simulink model "testDirect"  (Simulink 7.6)
settings: Solver=ode45  StartTime=0.0  StopTime=1400.0  RelTol=1e-3
libraries: xbsIndex_r4 x2187, casper_library x117, monroe_library x15, xps_library x1
(part 3 of 20)

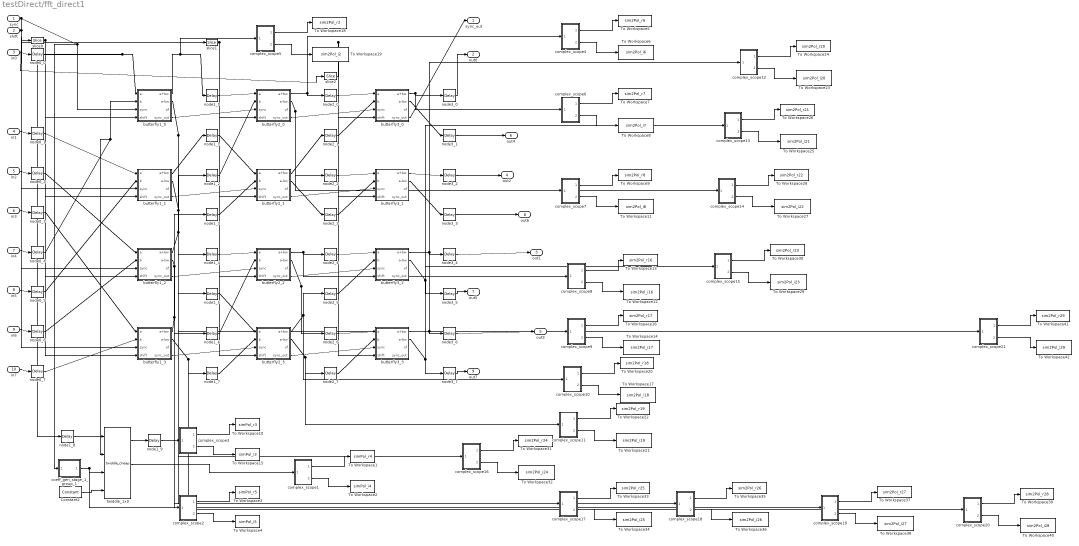
== system testDirect/fft_direct1 ==
  sync : Inport
  shift : Inport  Port=2
  in0 : Inport  Port=3
  in1 : Inport  Port=4
  in2 : Inport  Port=5
  in3 : Inport  Port=6
  in4 : Inport  Port=7
  in5 : Inport  Port=8
  in6 : Inport  Port=9
  in7 : Inport  Port=10
  Constant2 : xbsIndex_r4/Constant  arith_type=Signed (2's comp)  const=0.2  n_bits=18  bin_pt=17  explicit_period=on  period=1  equ=P=C  opselect=C  inp2=PCIN>>17  opr=+  inp1=P  carry=CIN  dbl_ovrd=off  block_type=constant
  To Workspace1 : ToWorkspace  VariableName=simPol_r4  SampleTime=-1  SaveFormat=Structure
  To Workspace10 : ToWorkspace  VariableName=simPol_r3  SampleTime=-1  SaveFormat=Structure
  To Workspace11 : ToWorkspace  VariableName=sim2Pol_i8  SampleTime=-1  SaveFormat=Structure
  To Workspace12 : ToWorkspace  VariableName=sim2Pol_i16  SampleTime=-1  SaveFormat=Structure
  To Workspace13 : ToWorkspace  VariableName=sim2Pol_r16  SampleTime=-1  SaveFormat=Structure
  To Workspace14 : ToWorkspace  VariableName=sim2Pol_i17  SampleTime=-1  SaveFormat=Structure
  To Workspace15 : ToWorkspace  VariableName=simPol_i3  SampleTime=-1  SaveFormat=Structure
  To Workspace16 : ToWorkspace  VariableName=sim2Pol_r17  SampleTime=-1  SaveFormat=Structure
  To Workspace17 : ToWorkspace  VariableName=sim2Pol_i18  SampleTime=-1  SaveFormat=Structure
  To Workspace18 : ToWorkspace  VariableName=sim2Pol_r2  SampleTime=-1  SaveFormat=Structure
  To Workspace19 : ToWorkspace  VariableName=sim2Pol_i2  SampleTime=-1  SaveFormat=Structure
  To Workspace2 : ToWorkspace  VariableName=simPol_i4  SampleTime=-1  SaveFormat=Structure
  To Workspace20 : ToWorkspace  VariableName=sim2Pol_r18  SampleTime=-1  SaveFormat=Structure
  To Workspace21 : ToWorkspace  VariableName=sim2Pol_i19  SampleTime=-1  SaveFormat=Structure
  To Workspace22 : ToWorkspace  VariableName=sim2Pol_r19  SampleTime=-1  SaveFormat=Structure
  To Workspace23 : ToWorkspace  VariableName=sim2Pol_i20  SampleTime=-1  SaveFormat=Structure
  To Workspace24 : ToWorkspace  VariableName=sim2Pol_r20  SampleTime=-1  SaveFormat=Structure
  To Workspace25 : ToWorkspace  VariableName=sim2Pol_i21  SampleTime=-1  SaveFormat=Structure
  To Workspace26 : ToWorkspace  VariableName=sim2Pol_r21  SampleTime=-1  SaveFormat=Structure
  To Workspace27 : ToWorkspace  VariableName=sim2Pol_i22  SampleTime=-1  SaveFormat=Structure
  To Workspace28 : ToWorkspace  VariableName=sim2Pol_r22  SampleTime=-1  SaveFormat=Structure
  To Workspace29 : ToWorkspace  VariableName=sim2Pol_i23  SampleTime=-1  SaveFormat=Structure
  To Workspace3 : ToWorkspace  VariableName=simPol_r5  SampleTime=-1  SaveFormat=Structure
  To Workspace30 : ToWorkspace  VariableName=sim2Pol_r23  SampleTime=-1  SaveFormat=Structure
  To Workspace31 : ToWorkspace  VariableName=sim2Pol_r24  SampleTime=-1  SaveFormat=Structure
  To Workspace32 : ToWorkspace  VariableName=sim2Pol_i24  SampleTime=-1  SaveFormat=Structure
  To Workspace33 : ToWorkspace  VariableName=sim2Pol_r25  SampleTime=-1  SaveFormat=Structure
  To Workspace34 : ToWorkspace  VariableName=sim2Pol_i25  SampleTime=-1  SaveFormat=Structure
  To Workspace35 : ToWorkspace  VariableName=sim2Pol_r26  SampleTime=-1  SaveFormat=Structure
  To Workspace36 : ToWorkspace  VariableName=sim2Pol_i26  SampleTime=-1  SaveFormat=Structure
  To Workspace37 : ToWorkspace  VariableName=sim2Pol_r27  SampleTime=-1  SaveFormat=Structure
  To Workspace38 : ToWorkspace  VariableName=sim2Pol_i27  SampleTime=-1  SaveFormat=Structure
  To Workspace39 : ToWorkspace  VariableName=sim2Pol_r28  SampleTime=-1  SaveFormat=Structure
  To Workspace4 : ToWorkspace  VariableName=simPol_i5  SampleTime=-1  SaveFormat=Structure
  To Workspace40 : ToWorkspace  VariableName=sim2Pol_i28  SampleTime=-1  SaveFormat=Structure
  To Workspace41 : ToWorkspace  VariableName=sim2Pol_r29  SampleTime=-1  SaveFormat=Structure
  To Workspace42 : ToWorkspace  VariableName=sim2Pol_i29  SampleTime=-1  SaveFormat=Structure
  To Workspace5 : ToWorkspace  VariableName=sim2Pol_r6  SampleTime=-1  SaveFormat=Structure
  To Workspace6 : ToWorkspace  VariableName=sim2Pol_i6  SampleTime=-1  SaveFormat=Structure
  To Workspace7 : ToWorkspace  VariableName=sim2Pol_r7  SampleTime=-1  SaveFormat=Structure
  To Workspace8 : ToWorkspace  VariableName=sim2Pol_i7  SampleTime=-1  SaveFormat=Structure
  To Workspace9 : ToWorkspace  VariableName=sim2Pol_r8  SampleTime=-1  SaveFormat=Structure
  butterfly1_0 : Subsystem  mask(butterfly_direct){biplex=off, FFTSize=8, Coeffs=getfield( getfield( get_param( gcb,'UserData' ), 'parameters'),'Coeffs'), coeff_bit_width=18, StepPeriod=0, bram_latency=2, coeffs_bram=on, input_bit_width=18, add_latency=2, mult_latency=3, quantization=Truncate, overflow=Wrap, arch=Virtex5, opt_target=Logic, use_hdl=on, use_embedded=off, dsp48_adders=on, shift=1, output_bit_width=18}
      mask prompts: biplex "Used in biplex FFT"; FFTSize "Size of FFT: (2^?)"; Coeffs "Coefficients: (0 to 2^(FFTSize-1)-1)"; coeff_bit_width "Coefficient Bit Width"; StepPeriod "Coefficient Step Period: (2^?)"; bram_latency "BRAM Latency"; coeffs_bram "Implement coefficients in BRAM"; input_bit_width "Input/Output Bit Width:"; add_latency "Add Latency"; mult_latency "Mult Latency"; quantization "Quantization Behavior"; overflow "Overflow Behavior"; arch "Architecture"; opt_target "Optimisation target"; use_hdl "Use behavioural HDL for multipliers"; use_embedded "Use embedded multipliers"; dsp48_adders "Use DSP48s for adders"; shift "Downshift binary point? (1 or 0)"; output_bit_width "Output Bit Width"
      mask help:
      | implements adders efficiently using 2 dsp48e slices, manually instatiated
      |
      | Update Log:
      | 08/05, 5:45 PM PST: inputs and outputs are registered
      | 08/24, 23:22 PST: 'shift' hard coded
      mask init:
      | butterfly_direct_init(gcb,...
      |               'biplex', biplex,...
      |               'FFTSize', FFTSize,...
      |               'Coeffs', Coeffs,...
      |               'coeff_bit_width', coeff_bit_width,...
      |               'StepPeriod', StepPeriod,...
      |               'bram_latency', bram_latency,...
      |               'coeffs_bram', coeffs_bram,...
      |               'input_bit_width', input_bit_width,...
      |               'output_bit_width', output_bit_width,...
      |               'add_latency', add_latency,...
      |               'mult_latency', mult_latency,...
      |               'quantization', quantization,...
      |               'overflow', overflow,...
      |               'arch', arch,...
      |               'opt_target', opt_target,...
      |               'use_hdl', use_hdl,...
      |               'use_embedded', use_embedded,...
      |               'dsp48_adders', dsp48_adders);
      settings: AttributesFormatString=FFTSize=8, coeff_bit_width=18
      mask StyleString: checkbox,edit,edit,edit,edit,edit,checkbox,edit,edit,edit,popup(Truncate|Round  (unbiased: +/- Inf)|Round  (unbiased: Even Values)),popup(Wrap|Saturate|Error),popup(Virtex2Pro|Virtex5),popup(Logic|Multipliers),checkbox,checkbox,checkbox,edit,edit
      mask CallbackString:
      | ||coeffs = eval(get_param(gcb,'Coeffs'));
      | visibs = get_param(gcb,'MaskVisibilities');
      | if length(coeffs) == 1,
      |     visibs{5} = 'off'; visibs{6} = 'off';
      |     visibs{7} = 'off';
      | else
      |     visibs{5} = 'on';
      |     visibs{6} = 'on'; visibs{7} = 'on';
      | end
      | set_param(gcb, 'MaskVisibilities', visibs);||||||||||||use_hdl = get_param(gcb,'use_hdl');
      | visibs = get_param(gcb, 'MaskVisibilities');
      | if( strcmp(use_hdl,'on')),
      |     set_param(gcb, 'use_embedded','off');
      |     visibs{16} = 'off';
      | else,
      |     visibs{16} = 'on';
      | end
      |     set_param(gcb, 'MaskVisibilities',visibs);||||
  butterfly1_1 : Subsystem  mask(butterfly_direct){biplex=off, FFTSize=8, Coeffs=getfield( getfield( get_param( gcb,'UserData' ), 'parameters'),'Coeffs'), coeff_bit_width=18, StepPeriod=0, bram_latency=2, coeffs_bram=on, input_bit_width=18, add_latency=2, mult_latency=3, quantization=Truncate, overflow=Wrap, arch=Virtex5, opt_target=Logic, use_hdl=on, use_embedded=off, dsp48_adders=on, shift=1, output_bit_width=18}
      mask prompts: biplex "Used in biplex FFT"; FFTSize "Size of FFT: (2^?)"; Coeffs "Coefficients: (0 to 2^(FFTSize-1)-1)"; coeff_bit_width "Coefficient Bit Width"; StepPeriod "Coefficient Step Period: (2^?)"; bram_latency "BRAM Latency"; coeffs_bram "Implement coefficients in BRAM"; input_bit_width "Input/Output Bit Width:"; add_latency "Add Latency"; mult_latency "Mult Latency"; quantization "Quantization Behavior"; overflow "Overflow Behavior"; arch "Architecture"; opt_target "Optimisation target"; use_hdl "Use behavioural HDL for multipliers"; use_embedded "Use embedded multipliers"; dsp48_adders "Use DSP48s for adders"; shift "Downshift binary point? (1 or 0)"; output_bit_width "Output Bit Width"
      mask help:
      | implements adders efficiently using 2 dsp48e slices, manually instatiated
      |
      | Update Log:
      | 08/05, 5:45 PM PST: inputs and outputs are registered
      | 08/24, 23:22 PST: 'shift' hard coded
      mask init: same as testDirect/fft_direct1/butterfly1_0
      settings: AttributesFormatString=FFTSize=8, coeff_bit_width=18
      mask StyleString: checkbox,edit,edit,edit,edit,edit,checkbox,edit,edit,edit,popup(Truncate|Round  (unbiased: +/- Inf)|Round  (unbiased: Even Values)),popup(Wrap|Saturate|Error),popup(Virtex2Pro|Virtex5),popup(Logic|Multipliers),checkbox,checkbox,checkbox,edit,edit
      mask CallbackString:
      | ||coeffs = eval(get_param(gcb,'Coeffs'));
      | visibs = get_param(gcb,'MaskVisibilities');
      | if length(coeffs) == 1,
      |     visibs{5} = 'off'; visibs{6} = 'off';
      |     visibs{7} = 'off';
      | else
      |     visibs{5} = 'on';
      |     visibs{6} = 'on'; visibs{7} = 'on';
      | end
      | set_param(gcb, 'MaskVisibilities', visibs);||||||||||||use_hdl = get_param(gcb,'use_hdl');
      | visibs = get_param(gcb, 'MaskVisibilities');
      | if( strcmp(use_hdl,'on')),
      |     set_param(gcb, 'use_embedded','off');
      |     visibs{16} = 'off';
      | else,
      |     visibs{16} = 'on';
      | end
      |     set_param(gcb, 'MaskVisibilities',visibs);||||
  butterfly1_2 : Subsystem  mask(butterfly_direct){biplex=off, FFTSize=8, Coeffs=getfield( getfield( get_param( gcb,'UserData' ), 'parameters'),'Coeffs'), coeff_bit_width=18, StepPeriod=0, bram_latency=2, coeffs_bram=on, input_bit_width=18, add_latency=2, mult_latency=3, quantization=Truncate, overflow=Wrap, arch=Virtex5, opt_target=Logic, use_hdl=on, use_embedded=off, dsp48_adders=on, shift=1, output_bit_width=18}
      mask prompts: biplex "Used in biplex FFT"; FFTSize "Size of FFT: (2^?)"; Coeffs "Coefficients: (0 to 2^(FFTSize-1)-1)"; coeff_bit_width "Coefficient Bit Width"; StepPeriod "Coefficient Step Period: (2^?)"; bram_latency "BRAM Latency"; coeffs_bram "Implement coefficients in BRAM"; input_bit_width "Input/Output Bit Width:"; add_latency "Add Latency"; mult_latency "Mult Latency"; quantization "Quantization Behavior"; overflow "Overflow Behavior"; arch "Architecture"; opt_target "Optimisation target"; use_hdl "Use behavioural HDL for multipliers"; use_embedded "Use embedded multipliers"; dsp48_adders "Use DSP48s for adders"; shift "Downshift binary point? (1 or 0)"; output_bit_width "Output Bit Width"
      mask help:
      | implements adders efficiently using 2 dsp48e slices, manually instatiated
      |
      | Update Log:
      | 08/05, 5:45 PM PST: inputs and outputs are registered
      | 08/24, 23:22 PST: 'shift' hard coded
      mask init: same as testDirect/fft_direct1/butterfly1_0
      settings: AttributesFormatString=FFTSize=8, coeff_bit_width=18
      mask StyleString: checkbox,edit,edit,edit,edit,edit,checkbox,edit,edit,edit,popup(Truncate|Round  (unbiased: +/- Inf)|Round  (unbiased: Even Values)),popup(Wrap|Saturate|Error),popup(Virtex2Pro|Virtex5),popup(Logic|Multipliers),checkbox,checkbox,checkbox,edit,edit
      mask CallbackString:
      | ||coeffs = eval(get_param(gcb,'Coeffs'));
      | visibs = get_param(gcb,'MaskVisibilities');
      | if length(coeffs) == 1,
      |     visibs{5} = 'off'; visibs{6} = 'off';
      |     visibs{7} = 'off';
      | else
      |     visibs{5} = 'on';
      |     visibs{6} = 'on'; visibs{7} = 'on';
      | end
      | set_param(gcb, 'MaskVisibilities', visibs);||||||||||||use_hdl = get_param(gcb,'use_hdl');
      | visibs = get_param(gcb, 'MaskVisibilities');
      | if( strcmp(use_hdl,'on')),
      |     set_param(gcb, 'use_embedded','off');
      |     visibs{16} = 'off';
      | else,
      |     visibs{16} = 'on';
      | end
      |     set_param(gcb, 'MaskVisibilities',visibs);||||
  butterfly1_3 : Subsystem  mask(butterfly_direct){biplex=off, FFTSize=8, Coeffs=getfield( getfield( get_param( gcb,'UserData' ), 'parameters'),'Coeffs'), coeff_bit_width=18, StepPeriod=0, bram_latency=2, coeffs_bram=on, input_bit_width=18, add_latency=2, mult_latency=3, quantization=Truncate, overflow=Wrap, arch=Virtex5, opt_target=Logic, use_hdl=on, use_embedded=off, dsp48_adders=on, shift=1, output_bit_width=18}
      mask prompts: biplex "Used in biplex FFT"; FFTSize "Size of FFT: (2^?)"; Coeffs "Coefficients: (0 to 2^(FFTSize-1)-1)"; coeff_bit_width "Coefficient Bit Width"; StepPeriod "Coefficient Step Period: (2^?)"; bram_latency "BRAM Latency"; coeffs_bram "Implement coefficients in BRAM"; input_bit_width "Input/Output Bit Width:"; add_latency "Add Latency"; mult_latency "Mult Latency"; quantization "Quantization Behavior"; overflow "Overflow Behavior"; arch "Architecture"; opt_target "Optimisation target"; use_hdl "Use behavioural HDL for multipliers"; use_embedded "Use embedded multipliers"; dsp48_adders "Use DSP48s for adders"; shift "Downshift binary point? (1 or 0)"; output_bit_width "Output Bit Width"
      mask help:
      | implements adders efficiently using 2 dsp48e slices, manually instatiated
      |
      | Update Log:
      | 08/05, 5:45 PM PST: inputs and outputs are registered
      | 08/24, 23:22 PST: 'shift' hard coded
      mask init: same as testDirect/fft_direct1/butterfly1_0
      settings: AttributesFormatString=FFTSize=8, coeff_bit_width=18
      mask StyleString: checkbox,edit,edit,edit,edit,edit,checkbox,edit,edit,edit,popup(Truncate|Round  (unbiased: +/- Inf)|Round  (unbiased: Even Values)),popup(Wrap|Saturate|Error),popup(Virtex2Pro|Virtex5),popup(Logic|Multipliers),checkbox,checkbox,checkbox,edit,edit
      mask CallbackString:
      | ||coeffs = eval(get_param(gcb,'Coeffs'));
      | visibs = get_param(gcb,'MaskVisibilities');
      | if length(coeffs) == 1,
      |     visibs{5} = 'off'; visibs{6} = 'off';
      |     visibs{7} = 'off';
      | else
      |     visibs{5} = 'on';
      |     visibs{6} = 'on'; visibs{7} = 'on';
      | end
      | set_param(gcb, 'MaskVisibilities', visibs);||||||||||||use_hdl = get_param(gcb,'use_hdl');
      | visibs = get_param(gcb, 'MaskVisibilities');
      | if( strcmp(use_hdl,'on')),
      |     set_param(gcb, 'use_embedded','off');
      |     visibs{16} = 'off';
      | else,
      |     visibs{16} = 'on';
      | end
      |     set_param(gcb, 'MaskVisibilities',visibs);||||
  butterfly2_0 : Subsystem  mask(butterfly_direct){biplex=off, FFTSize=8, Coeffs=getfield( getfield( get_param( gcb,'UserData' ), 'parameters'),'Coeffs'), coeff_bit_width=18, StepPeriod=0, bram_latency=2, coeffs_bram=on, input_bit_width=18, add_latency=2, mult_latency=3, quantization=Truncate, overflow=Wrap, arch=Virtex5, opt_target=Logic, use_hdl=on, use_embedded=off, dsp48_adders=on, shift=1, output_bit_width=18}
      mask prompts: biplex "Used in biplex FFT"; FFTSize "Size of FFT: (2^?)"; Coeffs "Coefficients: (0 to 2^(FFTSize-1)-1)"; coeff_bit_width "Coefficient Bit Width"; StepPeriod "Coefficient Step Period: (2^?)"; bram_latency "BRAM Latency"; coeffs_bram "Implement coefficients in BRAM"; input_bit_width "Input/Output Bit Width:"; add_latency "Add Latency"; mult_latency "Mult Latency"; quantization "Quantization Behavior"; overflow "Overflow Behavior"; arch "Architecture"; opt_target "Optimisation target"; use_hdl "Use behavioural HDL for multipliers"; use_embedded "Use embedded multipliers"; dsp48_adders "Use DSP48s for adders"; shift "Downshift binary point? (1 or 0)"; output_bit_width "Output Bit Width"
      mask help:
      | implements adders efficiently using 2 dsp48e slices, manually instatiated
      |
      | Update Log:
      | 08/05, 5:45 PM PST: inputs and outputs are registered
      | 08/24, 23:22 PST: 'shift' hard coded
      mask init: same as testDirect/fft_direct1/butterfly1_0
      settings: AttributesFormatString=FFTSize=8, coeff_bit_width=18
      mask StyleString: checkbox,edit,edit,edit,edit,edit,checkbox,edit,edit,edit,popup(Truncate|Round  (unbiased: +/- Inf)|Round  (unbiased: Even Values)),popup(Wrap|Saturate|Error),popup(Virtex2Pro|Virtex5),popup(Logic|Multipliers),checkbox,checkbox,checkbox,edit,edit
      mask CallbackString:
      | ||coeffs = eval(get_param(gcb,'Coeffs'));
      | visibs = get_param(gcb,'MaskVisibilities');
      | if length(coeffs) == 1,
      |     visibs{5} = 'off'; visibs{6} = 'off';
      |     visibs{7} = 'off';
      | else
      |     visibs{5} = 'on';
      |     visibs{6} = 'on'; visibs{7} = 'on';
      | end
      | set_param(gcb, 'MaskVisibilities', visibs);||||||||||||use_hdl = get_param(gcb,'use_hdl');
      | visibs = get_param(gcb, 'MaskVisibilities');
      | if( strcmp(use_hdl,'on')),
      |     set_param(gcb, 'use_embedded','off');
      |     visibs{16} = 'off';
      | else,
      |     visibs{16} = 'on';
      | end
      |     set_param(gcb, 'MaskVisibilities',visibs);||||
  butterfly2_1 : Subsystem  mask(butterfly_direct){biplex=off, FFTSize=8, Coeffs=getfield( getfield( get_param( gcb,'UserData' ), 'parameters'),'Coeffs'), coeff_bit_width=18, StepPeriod=0, bram_latency=2, coeffs_bram=on, input_bit_width=18, add_latency=2, mult_latency=3, quantization=Truncate, overflow=Wrap, arch=Virtex5, opt_target=Logic, use_hdl=on, use_embedded=off, dsp48_adders=on, shift=1, output_bit_width=18}
      mask prompts: biplex "Used in biplex FFT"; FFTSize "Size of FFT: (2^?)"; Coeffs "Coefficients: (0 to 2^(FFTSize-1)-1)"; coeff_bit_width "Coefficient Bit Width"; StepPeriod "Coefficient Step Period: (2^?)"; bram_latency "BRAM Latency"; coeffs_bram "Implement coefficients in BRAM"; input_bit_width "Input/Output Bit Width:"; add_latency "Add Latency"; mult_latency "Mult Latency"; quantization "Quantization Behavior"; overflow "Overflow Behavior"; arch "Architecture"; opt_target "Optimisation target"; use_hdl "Use behavioural HDL for multipliers"; use_embedded "Use embedded multipliers"; dsp48_adders "Use DSP48s for adders"; shift "Downshift binary point? (1 or 0)"; output_bit_width "Output Bit Width"
      mask help:
      | implements adders efficiently using 2 dsp48e slices, manually instatiated
      |
      | Update Log:
      | 08/05, 5:45 PM PST: inputs and outputs are registered
      | 08/24, 23:22 PST: 'shift' hard coded
      mask init: same as testDirect/fft_direct1/butterfly1_0
      settings: AttributesFormatString=FFTSize=8, coeff_bit_width=18
      mask StyleString: checkbox,edit,edit,edit,edit,edit,checkbox,edit,edit,edit,popup(Truncate|Round  (unbiased: +/- Inf)|Round  (unbiased: Even Values)),popup(Wrap|Saturate|Error),popup(Virtex2Pro|Virtex5),popup(Logic|Multipliers),checkbox,checkbox,checkbox,edit,edit
      mask CallbackString:
      | ||coeffs = eval(get_param(gcb,'Coeffs'));
      | visibs = get_param(gcb,'MaskVisibilities');
      | if length(coeffs) == 1,
      |     visibs{5} = 'off'; visibs{6} = 'off';
      |     visibs{7} = 'off';
      | else
      |     visibs{5} = 'on';
      |     visibs{6} = 'on'; visibs{7} = 'on';
      | end
      | set_param(gcb, 'MaskVisibilities', visibs);||||||||||||use_hdl = get_param(gcb,'use_hdl');
      | visibs = get_param(gcb, 'MaskVisibilities');
      | if( strcmp(use_hdl,'on')),
      |     set_param(gcb, 'use_embedded','off');
      |     visibs{16} = 'off';
      | else,
      |     visibs{16} = 'on';
      | end
      |     set_param(gcb, 'MaskVisibilities',visibs);||||
  butterfly2_2 : Subsystem  mask(butterfly_direct){biplex=off, FFTSize=8, Coeffs=getfield( getfield( get_param( gcb,'UserData' ), 'parameters'),'Coeffs'), coeff_bit_width=18, StepPeriod=0, bram_latency=2, coeffs_bram=on, input_bit_width=18, add_latency=2, mult_latency=3, quantization=Truncate, overflow=Wrap, arch=Virtex5, opt_target=Logic, use_hdl=on, use_embedded=off, dsp48_adders=on, shift=1, output_bit_width=18}
      mask prompts: biplex "Used in biplex FFT"; FFTSize "Size of FFT: (2^?)"; Coeffs "Coefficients: (0 to 2^(FFTSize-1)-1)"; coeff_bit_width "Coefficient Bit Width"; StepPeriod "Coefficient Step Period: (2^?)"; bram_latency "BRAM Latency"; coeffs_bram "Implement coefficients in BRAM"; input_bit_width "Input/Output Bit Width:"; add_latency "Add Latency"; mult_latency "Mult Latency"; quantization "Quantization Behavior"; overflow "Overflow Behavior"; arch "Architecture"; opt_target "Optimisation target"; use_hdl "Use behavioural HDL for multipliers"; use_embedded "Use embedded multipliers"; dsp48_adders "Use DSP48s for adders"; shift "Downshift binary point? (1 or 0)"; output_bit_width "Output Bit Width"
      mask help:
      | implements adders efficiently using 2 dsp48e slices, manually instatiated
      |
      | Update Log:
      | 08/05, 5:45 PM PST: inputs and outputs are registered
      | 08/24, 23:22 PST: 'shift' hard coded
      mask init: same as testDirect/fft_direct1/butterfly1_0
      settings: AttributesFormatString=FFTSize=8, coeff_bit_width=18
      mask StyleString: checkbox,edit,edit,edit,edit,edit,checkbox,edit,edit,edit,popup(Truncate|Round  (unbiased: +/- Inf)|Round  (unbiased: Even Values)),popup(Wrap|Saturate|Error),popup(Virtex2Pro|Virtex5),popup(Logic|Multipliers),checkbox,checkbox,checkbox,edit,edit
      mask CallbackString:
      | ||coeffs = eval(get_param(gcb,'Coeffs'));
      | visibs = get_param(gcb,'MaskVisibilities');
      | if length(coeffs) == 1,
      |     visibs{5} = 'off'; visibs{6} = 'off';
      |     visibs{7} = 'off';
      | else
      |     visibs{5} = 'on';
      |     visibs{6} = 'on'; visibs{7} = 'on';
      | end
      | set_param(gcb, 'MaskVisibilities', visibs);||||||||||||use_hdl = get_param(gcb,'use_hdl');
      | visibs = get_param(gcb, 'MaskVisibilities');
      | if( strcmp(use_hdl,'on')),
      |     set_param(gcb, 'use_embedded','off');
      |     visibs{16} = 'off';
      | else,
      |     visibs{16} = 'on';
      | end
      |     set_param(gcb, 'MaskVisibilities',visibs);||||
  butterfly2_3 : Subsystem  mask(butterfly_direct){biplex=off, FFTSize=8, Coeffs=getfield( getfield( get_param( gcb,'UserData' ), 'parameters'),'Coeffs'), coeff_bit_width=18, StepPeriod=0, bram_latency=2, coeffs_bram=on, input_bit_width=18, add_latency=2, mult_latency=3, quantization=Truncate, overflow=Wrap, arch=Virtex5, opt_target=Logic, use_hdl=on, use_embedded=off, dsp48_adders=on, shift=1, output_bit_width=18}
      mask prompts: biplex "Used in biplex FFT"; FFTSize "Size of FFT: (2^?)"; Coeffs "Coefficients: (0 to 2^(FFTSize-1)-1)"; coeff_bit_width "Coefficient Bit Width"; StepPeriod "Coefficient Step Period: (2^?)"; bram_latency "BRAM Latency"; coeffs_bram "Implement coefficients in BRAM"; input_bit_width "Input/Output Bit Width:"; add_latency "Add Latency"; mult_latency "Mult Latency"; quantization "Quantization Behavior"; overflow "Overflow Behavior"; arch "Architecture"; opt_target "Optimisation target"; use_hdl "Use behavioural HDL for multipliers"; use_embedded "Use embedded multipliers"; dsp48_adders "Use DSP48s for adders"; shift "Downshift binary point? (1 or 0)"; output_bit_width "Output Bit Width"
      mask help:
      | implements adders efficiently using 2 dsp48e slices, manually instatiated
      |
      | Update Log:
      | 08/05, 5:45 PM PST: inputs and outputs are registered
      | 08/24, 23:22 PST: 'shift' hard coded
      mask init: same as testDirect/fft_direct1/butterfly1_0
      settings: AttributesFormatString=FFTSize=8, coeff_bit_width=18
      mask StyleString: checkbox,edit,edit,edit,edit,edit,checkbox,edit,edit,edit,popup(Truncate|Round  (unbiased: +/- Inf)|Round  (unbiased: Even Values)),popup(Wrap|Saturate|Error),popup(Virtex2Pro|Virtex5),popup(Logic|Multipliers),checkbox,checkbox,checkbox,edit,edit
      mask CallbackString:
      | ||coeffs = eval(get_param(gcb,'Coeffs'));
      | visibs = get_param(gcb,'MaskVisibilities');
      | if length(coeffs) == 1,
      |     visibs{5} = 'off'; visibs{6} = 'off';
      |     visibs{7} = 'off';
      | else
      |     visibs{5} = 'on';
      |     visibs{6} = 'on'; visibs{7} = 'on';
      | end
      | set_param(gcb, 'MaskVisibilities', visibs);||||||||||||use_hdl = get_param(gcb,'use_hdl');
      | visibs = get_param(gcb, 'MaskVisibilities');
      | if( strcmp(use_hdl,'on')),
      |     set_param(gcb, 'use_embedded','off');
      |     visibs{16} = 'off';
      | else,
      |     visibs{16} = 'on';
      | end
      |     set_param(gcb, 'MaskVisibilities',visibs);||||
  butterfly3_0 : Subsystem  mask(butterfly_direct){biplex=off, FFTSize=8, Coeffs=getfield( getfield( get_param( gcb,'UserData' ), 'parameters'),'Coeffs'), coeff_bit_width=18, StepPeriod=0, bram_latency=2, coeffs_bram=on, input_bit_width=18, add_latency=2, mult_latency=3, quantization=Truncate, overflow=Wrap, arch=Virtex5, opt_target=Logic, use_hdl=on, use_embedded=off, dsp48_adders=on, shift=1, output_bit_width=18}
      mask prompts: biplex "Used in biplex FFT"; FFTSize "Size of FFT: (2^?)"; Coeffs "Coefficients: (0 to 2^(FFTSize-1)-1)"; coeff_bit_width "Coefficient Bit Width"; StepPeriod "Coefficient Step Period: (2^?)"; bram_latency "BRAM Latency"; coeffs_bram "Implement coefficients in BRAM"; input_bit_width "Input/Output Bit Width:"; add_latency "Add Latency"; mult_latency "Mult Latency"; quantization "Quantization Behavior"; overflow "Overflow Behavior"; arch "Architecture"; opt_target "Optimisation target"; use_hdl "Use behavioural HDL for multipliers"; use_embedded "Use embedded multipliers"; dsp48_adders "Use DSP48s for adders"; shift "Downshift binary point? (1 or 0)"; output_bit_width "Output Bit Width"
      mask help:
      | implements adders efficiently using 2 dsp48e slices, manually instatiated
      |
      | Update Log:
      | 08/05, 5:45 PM PST: inputs and outputs are registered
      | 08/24, 23:22 PST: 'shift' hard coded
      mask init: same as testDirect/fft_direct1/butterfly1_0
      settings: AttributesFormatString=FFTSize=8, coeff_bit_width=18
      mask StyleString: checkbox,edit,edit,edit,edit,edit,checkbox,edit,edit,edit,popup(Truncate|Round  (unbiased: +/- Inf)|Round  (unbiased: Even Values)),popup(Wrap|Saturate|Error),popup(Virtex2Pro|Virtex5),popup(Logic|Multipliers),checkbox,checkbox,checkbox,edit,edit
      mask CallbackString:
      | ||coeffs = eval(get_param(gcb,'Coeffs'));
      | visibs = get_param(gcb,'MaskVisibilities');
      | if length(coeffs) == 1,
      |     visibs{5} = 'off'; visibs{6} = 'off';
      |     visibs{7} = 'off';
      | else
      |     visibs{5} = 'on';
      |     visibs{6} = 'on'; visibs{7} = 'on';
      | end
      | set_param(gcb, 'MaskVisibilities', visibs);||||||||||||use_hdl = get_param(gcb,'use_hdl');
      | visibs = get_param(gcb, 'MaskVisibilities');
      | if( strcmp(use_hdl,'on')),
      |     set_param(gcb, 'use_embedded','off');
      |     visibs{16} = 'off';
      | else,
      |     visibs{16} = 'on';
      | end
      |     set_param(gcb, 'MaskVisibilities',visibs);||||
  butterfly3_1 : Subsystem  mask(butterfly_direct){biplex=off, FFTSize=8, Coeffs=getfield( getfield( get_param( gcb,'UserData' ), 'parameters'),'Coeffs'), coeff_bit_width=18, StepPeriod=0, bram_latency=2, coeffs_bram=on, input_bit_width=18, add_latency=2, mult_latency=3, quantization=Truncate, overflow=Wrap, arch=Virtex5, opt_target=Logic, use_hdl=on, use_embedded=off, dsp48_adders=on, shift=1, output_bit_width=18}
      mask prompts: biplex "Used in biplex FFT"; FFTSize "Size of FFT: (2^?)"; Coeffs "Coefficients: (0 to 2^(FFTSize-1)-1)"; coeff_bit_width "Coefficient Bit Width"; StepPeriod "Coefficient Step Period: (2^?)"; bram_latency "BRAM Latency"; coeffs_bram "Implement coefficients in BRAM"; input_bit_width "Input/Output Bit Width:"; add_latency "Add Latency"; mult_latency "Mult Latency"; quantization "Quantization Behavior"; overflow "Overflow Behavior"; arch "Architecture"; opt_target "Optimisation target"; use_hdl "Use behavioural HDL for multipliers"; use_embedded "Use embedded multipliers"; dsp48_adders "Use DSP48s for adders"; shift "Downshift binary point? (1 or 0)"; output_bit_width "Output Bit Width"
      mask help:
      | implements adders efficiently using 2 dsp48e slices, manually instatiated
      |
      | Update Log:
      | 08/05, 5:45 PM PST: inputs and outputs are registered
      | 08/24, 23:22 PST: 'shift' hard coded
      mask init: same as testDirect/fft_direct1/butterfly1_0
      settings: AttributesFormatString=FFTSize=8, coeff_bit_width=18
      mask StyleString: checkbox,edit,edit,edit,edit,edit,checkbox,edit,edit,edit,popup(Truncate|Round  (unbiased: +/- Inf)|Round  (unbiased: Even Values)),popup(Wrap|Saturate|Error),popup(Virtex2Pro|Virtex5),popup(Logic|Multipliers),checkbox,checkbox,checkbox,edit,edit
      mask CallbackString:
      | ||coeffs = eval(get_param(gcb,'Coeffs'));
      | visibs = get_param(gcb,'MaskVisibilities');
      | if length(coeffs) == 1,
      |     visibs{5} = 'off'; visibs{6} = 'off';
      |     visibs{7} = 'off';
      | else
      |     visibs{5} = 'on';
      |     visibs{6} = 'on'; visibs{7} = 'on';
      | end
      | set_param(gcb, 'MaskVisibilities', visibs);||||||||||||use_hdl = get_param(gcb,'use_hdl');
      | visibs = get_param(gcb, 'MaskVisibilities');
      | if( strcmp(use_hdl,'on')),
      |     set_param(gcb, 'use_embedded','off');
      |     visibs{16} = 'off';
      | else,
      |     visibs{16} = 'on';
      | end
      |     set_param(gcb, 'MaskVisibilities',visibs);||||
  butterfly3_2 : Subsystem  mask(butterfly_direct){biplex=off, FFTSize=8, Coeffs=getfield( getfield( get_param( gcb,'UserData' ), 'parameters'),'Coeffs'), coeff_bit_width=18, StepPeriod=0, bram_latency=2, coeffs_bram=on, input_bit_width=18, add_latency=2, mult_latency=3, quantization=Truncate, overflow=Wrap, arch=Virtex5, opt_target=Logic, use_hdl=on, use_embedded=off, dsp48_adders=on, shift=1, output_bit_width=18}
      mask prompts: biplex "Used in biplex FFT"; FFTSize "Size of FFT: (2^?)"; Coeffs "Coefficients: (0 to 2^(FFTSize-1)-1)"; coeff_bit_width "Coefficient Bit Width"; StepPeriod "Coefficient Step Period: (2^?)"; bram_latency "BRAM Latency"; coeffs_bram "Implement coefficients in BRAM"; input_bit_width "Input/Output Bit Width:"; add_latency "Add Latency"; mult_latency "Mult Latency"; quantization "Quantization Behavior"; overflow "Overflow Behavior"; arch "Architecture"; opt_target "Optimisation target"; use_hdl "Use behavioural HDL for multipliers"; use_embedded "Use embedded multipliers"; dsp48_adders "Use DSP48s for adders"; shift "Downshift binary point? (1 or 0)"; output_bit_width "Output Bit Width"
      mask help:
      | implements adders efficiently using 2 dsp48e slices, manually instatiated
      |
      | Update Log:
      | 08/05, 5:45 PM PST: inputs and outputs are registered
      | 08/24, 23:22 PST: 'shift' hard coded
      mask init: same as testDirect/fft_direct1/butterfly1_0
      settings: AttributesFormatString=FFTSize=8, coeff_bit_width=18
      mask StyleString: checkbox,edit,edit,edit,edit,edit,checkbox,edit,edit,edit,popup(Truncate|Round  (unbiased: +/- Inf)|Round  (unbiased: Even Values)),popup(Wrap|Saturate|Error),popup(Virtex2Pro|Virtex5),popup(Logic|Multipliers),checkbox,checkbox,checkbox,edit,edit
      mask CallbackString:
      | ||coeffs = eval(get_param(gcb,'Coeffs'));
      | visibs = get_param(gcb,'MaskVisibilities');
      | if length(coeffs) == 1,
      |     visibs{5} = 'off'; visibs{6} = 'off';
      |     visibs{7} = 'off';
      | else
      |     visibs{5} = 'on';
      |     visibs{6} = 'on'; visibs{7} = 'on';
      | end
      | set_param(gcb, 'MaskVisibilities', visibs);||||||||||||use_hdl = get_param(gcb,'use_hdl');
      | visibs = get_param(gcb, 'MaskVisibilities');
      | if( strcmp(use_hdl,'on')),
      |     set_param(gcb, 'use_embedded','off');
      |     visibs{16} = 'off';
      | else,
      |     visibs{16} = 'on';
      | end
      |     set_param(gcb, 'MaskVisibilities',visibs);||||
  butterfly3_3 : Subsystem  mask(butterfly_direct){biplex=off, FFTSize=8, Coeffs=getfield( getfield( get_param( gcb,'UserData' ), 'parameters'),'Coeffs'), coeff_bit_width=18, StepPeriod=0, bram_latency=2, coeffs_bram=on, input_bit_width=18, add_latency=2, mult_latency=3, quantization=Truncate, overflow=Wrap, arch=Virtex5, opt_target=Logic, use_hdl=on, use_embedded=off, dsp48_adders=on, shift=1, output_bit_width=18}
      mask prompts: biplex "Used in biplex FFT"; FFTSize "Size of FFT: (2^?)"; Coeffs "Coefficients: (0 to 2^(FFTSize-1)-1)"; coeff_bit_width "Coefficient Bit Width"; StepPeriod "Coefficient Step Period: (2^?)"; bram_latency "BRAM Latency"; coeffs_bram "Implement coefficients in BRAM"; input_bit_width "Input/Output Bit Width:"; add_latency "Add Latency"; mult_latency "Mult Latency"; quantization "Quantization Behavior"; overflow "Overflow Behavior"; arch "Architecture"; opt_target "Optimisation target"; use_hdl "Use behavioural HDL for multipliers"; use_embedded "Use embedded multipliers"; dsp48_adders "Use DSP48s for adders"; shift "Downshift binary point? (1 or 0)"; output_bit_width "Output Bit Width"
      mask help:
      | implements adders efficiently using 2 dsp48e slices, manually instatiated
      |
      | Update Log:
      | 08/05, 5:45 PM PST: inputs and outputs are registered
      | 08/24, 23:22 PST: 'shift' hard coded
      mask init: same as testDirect/fft_direct1/butterfly1_0
      settings: AttributesFormatString=FFTSize=8, coeff_bit_width=18
      mask StyleString: checkbox,edit,edit,edit,edit,edit,checkbox,edit,edit,edit,popup(Truncate|Round  (unbiased: +/- Inf)|Round  (unbiased: Even Values)),popup(Wrap|Saturate|Error),popup(Virtex2Pro|Virtex5),popup(Logic|Multipliers),checkbox,checkbox,checkbox,edit,edit
      mask CallbackString:
      | ||coeffs = eval(get_param(gcb,'Coeffs'));
      | visibs = get_param(gcb,'MaskVisibilities');
      | if length(coeffs) == 1,
      |     visibs{5} = 'off'; visibs{6} = 'off';
      |     visibs{7} = 'off';
      | else
      |     visibs{5} = 'on';
      |     visibs{6} = 'on'; visibs{7} = 'on';
      | end
      | set_param(gcb, 'MaskVisibilities', visibs);||||||||||||use_hdl = get_param(gcb,'use_hdl');
      | visibs = get_param(gcb, 'MaskVisibilities');
      | if( strcmp(use_hdl,'on')),
      |     set_param(gcb, 'use_embedded','off');
      |     visibs{16} = 'off';
      | else,
      |     visibs{16} = 'on';
      | end
      |     set_param(gcb, 'MaskVisibilities',visibs);||||
  coeff_gen_stage_1_group_1 : Subsystem
  complex_scope1 : Subsystem
  complex_scope10 : Subsystem
  complex_scope11 : Subsystem
  complex_scope12 : Subsystem
  complex_scope13 : Subsystem
  complex_scope14 : Subsystem
  complex_scope15 : Subsystem
  complex_scope16 : Subsystem
  complex_scope17 : Subsystem
  complex_scope18 : Subsystem
  complex_scope19 : Subsystem
  complex_scope2 : Subsystem
  complex_scope20 : Subsystem
  complex_scope21 : Subsystem
  complex_scope3 : Subsystem
  complex_scope4 : Subsystem
  complex_scope5 : Subsystem
  complex_scope6 : Subsystem
  complex_scope7 : Subsystem
  complex_scope8 : Subsystem
  complex_scope9 : Subsystem
  node0_{0..7} (8 alike) : xbsIndex_r4/Delay  en=off  latency=0  dbl_ovrd=off  reg_retiming=off  block_type=delay
      settings: block_version=11.4
  node1_{0..7} (8 alike) : xbsIndex_r4/Delay  en=off  latency=0  dbl_ovrd=off  reg_retiming=off  block_type=delay
      settings: block_version=11.4
  node1_8 : xbsIndex_r4/Delay  en=off  latency=1  dbl_ovrd=off  reg_retiming=off  block_type=delay
      settings: block_version=11.4
  node1_9 : xbsIndex_r4/Delay  en=off  latency=2  dbl_ovrd=off  reg_retiming=off  block_type=delay
      settings: block_version=11.4
  node2_{0..7} (8 alike) : xbsIndex_r4/Delay  en=off  latency=0  dbl_ovrd=off  reg_retiming=off  block_type=delay
      settings: block_version=11.4
  node3_{0..7} (8 alike) : xbsIndex_r4/Delay  en=off  latency=0  dbl_ovrd=off  reg_retiming=off  block_type=delay
      settings: block_version=11.4
  slice0 : xbsIndex_r4/Slice  nbits=1  boolean_output=on  mode=Lower Bit Location + Width  bit1=0  base1=MSB of Input  bit0=0  base0=LSB of Input  dbl_ovrd=off  block_type=slice
  slice1 : xbsIndex_r4/Slice  nbits=1  boolean_output=on  mode=Lower Bit Location + Width  bit1=0  base1=MSB of Input  bit0=1  base0=LSB of Input  dbl_ovrd=off  block_type=slice
  slice2 : xbsIndex_r4/Slice  nbits=1  boolean_output=on  mode=Lower Bit Location + Width  bit1=0  base1=MSB of Input  bit0=2  base0=LSB of Input  dbl_ovrd=off  block_type=slice
  twiddle_1x0 : monroe_library/twiddle_cheap  SystemSampleTime=-1  a_n_bits=18  a_bin_pt=17  b_n_bits=18  b_bin_pt=17  w_n_bits=18  w_bin_pt=17  a_delay=0  apbw_delay=0  out_n_bits=18  downshift_at_end=1
  sync_out : Outport
  out0 : Outport  Port=2
  out1 : Outport  Port=3
  out2 : Outport  Port=4
  out3 : Outport  Port=5
  out4 : Outport  Port=6
  out5 : Outport  Port=7
  out6 : Outport  Port=8
  out7 : Outport  Port=9
  wires:
  butterfly2_3/4(sync_out) -> butterfly3_3/3(sync)
  butterfly3_3/2(a-bw) -> node3_7/1, complex_scope15/1(In1)
  butterfly3_3/1(a+bw) -> node3_6/1, complex_scope14/1(In1)
  node2_7/1 -> butterfly3_3/2(b)
  node2_6/1 -> butterfly3_3/1(a)
  butterfly2_2/4(sync_out) -> butterfly3_2/3(sync)
  butterfly3_2/2(a-bw) -> node3_5/1, complex_scope13/1(In1)
  butterfly3_2/1(a+bw) -> node3_4/1, complex_scope12/1(In1)
  node2_5/1 -> butterfly3_2/2(b)
  node2_4/1 -> butterfly3_2/1(a)
  butterfly2_1/4(sync_out) -> butterfly3_1/3(sync)
  butterfly3_1/2(a-bw) -> node3_3/1
  butterfly3_1/1(a+bw) -> node3_2/1
  node2_3/1 -> butterfly3_1/2(b)
  node2_2/1 -> butterfly3_1/1(a)
  slice2/1 -> butterfly3_3/4(shift), butterfly3_2/4(shift), butterfly3_1/4(shift), butterfly3_0/4(shift)
  butterfly3_0/4(sync_out) -> sync_out/1
  butterfly2_0/4(sync_out) -> butterfly3_0/3(sync)
  butterfly3_0/2(a-bw) -> node3_1/1
  butterfly3_0/1(a+bw) -> node3_0/1, complex_scope6/1(In1)
  node2_1/1 -> butterfly3_0/2(b)
  node2_0/1 -> butterfly3_0/1(a)
  butterfly1_3/4(sync_out) -> butterfly2_3/3(sync)
  butterfly2_3/2(a-bw) -> node2_7/1, complex_scope11/1(In1)
  butterfly2_3/1(a+bw) -> node2_5/1, complex_scope10/1(In1)
  node1_7/1 -> butterfly2_3/2(b)
  node1_5/1 -> butterfly2_3/1(a)
  butterfly1_2/4(sync_out) -> butterfly2_2/3(sync)
  butterfly2_2/2(a-bw) -> node2_6/1, complex_scope9/1(In1)
  butterfly2_2/1(a+bw) -> node2_4/1, complex_scope8/1(In1)
  node1_6/1 -> butterfly2_2/2(b)
  node1_4/1 -> butterfly2_2/1(a)
  butterfly1_1/4(sync_out) -> butterfly2_1/3(sync)
  butterfly2_1/2(a-bw) -> node2_3/1
  butterfly2_1/1(a+bw) -> node2_1/1
  node1_3/1 -> butterfly2_1/2(b)
  node1_1/1 -> butterfly2_1/1(a)
  slice1/1 -> butterfly2_3/4(shift), butterfly2_2/4(shift), butterfly2_1/4(shift), butterfly2_0/4(shift)
  butterfly1_0/4(sync_out) -> butterfly2_0/3(sync)
  butterfly2_0/2(a-bw) -> node2_2/1, complex_scope7/1(In1)
  butterfly2_0/1(a+bw) -> node2_0/1, complex_scope4/1(In1)
  node1_2/1 -> butterfly2_0/2(b)
  node1_0/1 -> butterfly2_0/1(a)
  butterfly1_3/2(a-bw) -> node1_7/1, complex_scope20/1(In1)
  butterfly1_3/1(a+bw) -> node1_3/1, complex_scope19/1(In1)
  node0_7/1 -> butterfly1_3/2(b)
  node0_3/1 -> butterfly1_3/1(a)
  butterfly1_2/2(a-bw) -> node1_6/1, complex_scope18/1(In1)
  butterfly1_2/1(a+bw) -> node1_2/1, complex_scope17/1(In1)
  node0_6/1 -> butterfly1_2/2(b)
  node0_2/1 -> butterfly1_2/1(a)
  butterfly1_1/2(a-bw) -> complex_scope21/1(In1), node1_5/1
  butterfly1_1/1(a+bw) -> node1_1/1
  node0_5/1 -> butterfly1_1/2(b)
  node0_1/1 -> butterfly1_1/1(a)
  slice0/1 -> butterfly1_3/4(shift), butterfly1_2/4(shift), butterfly1_1/4(shift), butterfly1_0/4(shift)
  sync/1 -> butterfly1_3/3(sync), butterfly1_2/3(sync), butterfly1_1/3(sync), butterfly1_0/3(sync), coeff_gen_stage_1_group_1/1(sync)
  butterfly1_0/2(a-bw) -> node1_4/1, complex_scope16/1(In1)
  butterfly1_0/1(a+bw) -> node1_0/1, complex_scope5/1(In1)
  node0_4/1 -> butterfly1_0/2(b), twiddle_1x0/2
  node0_0/1 -> butterfly1_0/1(a), node1_8/1
  node3_7/1 -> out7/1
  node3_6/1 -> out3/1
  node3_5/1 -> out5/1
  node3_4/1 -> out1/1
  node3_3/1 -> out6/1
  node3_2/1 -> out2/1
  node3_1/1 -> out4/1
  node3_0/1 -> out0/1
  shift/1 -> slice2/1, slice1/1, slice0/1
  in7/1 -> node0_7/1
  in6/1 -> node0_6/1
  in5/1 -> node0_5/1
  in4/1 -> node0_4/1
  in3/1 -> node0_3/1
  in2/1 -> node0_2/1
  in1/1 -> node0_1/1
  in0/1 -> node0_0/1
  complex_scope5/1(Real) -> To Workspace18/1
  complex_scope5/2(Imag) -> To Workspace19/1
  node1_8/1 -> twiddle_1x0/1
  twiddle_1x0/1 -> node1_9/1
  complex_scope3/1(Real) -> To Workspace10/1
  complex_scope3/2(Imag) -> To Workspace15/1
  complex_scope1/1(Real) -> To Workspace1/1
  complex_scope1/2(Imag) -> To Workspace2/1
  node1_9/1 -> complex_scope3/1(In1)
  twiddle_1x0/2 -> complex_scope1/1(In1)
  coeff_gen_stage_1_group_1/1(coeff) -> twiddle_1x0/3, complex_scope2/1(In1)
  Constant2/1 -> twiddle_1x0/4
  complex_scope2/1(Real) -> To Workspace3/1
  complex_scope2/2(Imag) -> To Workspace4/1
  complex_scope4/1(Real) -> To Workspace5/1
  complex_scope4/2(Imag) -> To Workspace6/1
  complex_scope6/1(Real) -> To Workspace7/1
  complex_scope6/2(Imag) -> To Workspace8/1
  complex_scope7/1(Real) -> To Workspace9/1
  complex_scope7/2(Imag) -> To Workspace11/1
  complex_scope8/1(Real) -> To Workspace13/1
  complex_scope8/2(Imag) -> To Workspace12/1
  complex_scope9/1(Real) -> To Workspace16/1
  complex_scope9/2(Imag) -> To Workspace14/1
  complex_scope10/1(Real) -> To Workspace20/1
  complex_scope10/2(Imag) -> To Workspace17/1
  complex_scope11/1(Real) -> To Workspace22/1
  complex_scope11/2(Imag) -> To Workspace21/1
  complex_scope12/1(Real) -> To Workspace24/1
  complex_scope12/2(Imag) -> To Workspace23/1
  complex_scope13/1(Real) -> To Workspace26/1
  complex_scope13/2(Imag) -> To Workspace25/1
  complex_scope14/1(Real) -> To Workspace28/1
  complex_scope14/2(Imag) -> To Workspace27/1
  complex_scope15/1(Real) -> To Workspace30/1
  complex_scope15/2(Imag) -> To Workspace29/1
  complex_scope16/1(Real) -> To Workspace31/1
  complex_scope16/2(Imag) -> To Workspace32/1
  complex_scope17/1(Real) -> To Workspace33/1
  complex_scope17/2(Imag) -> To Workspace34/1
  complex_scope18/1(Real) -> To Workspace35/1
  complex_scope18/2(Imag) -> To Workspace36/1
  complex_scope19/1(Real) -> To Workspace37/1
  complex_scope19/2(Imag) -> To Workspace38/1
  complex_scope20/1(Real) -> To Workspace39/1
  complex_scope20/2(Imag) -> To Workspace40/1
  complex_scope21/1(Real) -> To Workspace41/1
  complex_scope21/2(Imag) -> To Workspace42/1

== system testDirect/oneSync ==
  Costant3 : xbsIndex_r4/Constant  arith_type=Unsigned  const=2^4 -1  n_bits=4  bin_pt=0  explicit_period=on  period=1  equ=P=C  opselect=C  inp2=PCIN>>17  opr=+  inp1=P  carry=CIN  dbl_ovrd=off  block_type=constant
      settings: block_version=11.4
  Counter1 : xbsIndex_r4/Counter  cnt_type=Free Running  cnt_to=2^11-2  operation=Up  start_count=0  cnt_by_val=1  arith_type=Unsigned  n_bits=4  bin_pt=0  load_pin=off  rst=off  en=off  explicit_period=on  period=1  dbl_ovrd=off  use_behavioral_HDL=off  implementation=Fabric  block_type=counter
      settings: block_version=11.3
  Inverter : xbsIndex_r4/Inverter  en=off  latency=0  dbl_ovrd=off  block_type=inv
      settings: block_version=11.4
  Logical : xbsIndex_r4/Logical  logical_function=OR  inputs=2  en=off  latency=0  precision=Full  arith_type=Unsigned  n_bits=16  bin_pt=0  align_bp=on  dbl_ovrd=off  block_type=logical
      settings: block_version=11.4
  Logical1 : xbsIndex_r4/Logical  logical_function=AND  inputs=2  en=off  latency=2  precision=Full  arith_type=Unsigned  n_bits=16  bin_pt=0  align_bp=on  dbl_ovrd=off  block_type=logical
      settings: block_version=11.4
  Relational1 : xbsIndex_r4/Relational  mode=a=b  en=off  latency=1  dbl_ovrd=off  block_type=relational
  hadSync : xbsIndex_r4/Register  init=0  rst=off  en=off  dbl_ovrd=off  block_type=register
      settings: block_version=11.4
  sync_out : Outport
  wires:
  Counter1/1 -> Relational1/1
  Costant3/1 -> Relational1/2
  Inverter/1 -> Logical1/1
  Relational1/1 -> Logical1/2
  Logical1/1 -> Logical/1, sync_out/1
  Logical/1 -> hadSync/1
  hadSync/1 -> Inverter/1, Logical/2

== system testDirect/fft_direct/bulk_delay_0_1 ==
  in1 : Inport
  in2 : Inport  Port=2
  in3 : Inport  Port=3
  in4 : Inport  Port=4
  in5 : Inport  Port=5
  in6 : Inport  Port=6
  in7 : Inport  Port=7
  in8 : Inport  Port=8
  delay_{1,3,5,7} (4 alike) : xbsIndex_r4/Delay  en=off  latency=7  dbl_ovrd=off  reg_retiming=off  block_type=delay
  delay_{2,4,6,8} (4 alike) : xbsIndex_r4/Delay  en=off  latency=6  dbl_ovrd=off  reg_retiming=off  block_type=delay
  out1 : Outport
  out2 : Outport  Port=2
  out3 : Outport  Port=3
  out4 : Outport  Port=4
  out5 : Outport  Port=5
  out6 : Outport  Port=6
  out7 : Outport  Port=7
  out8 : Outport  Port=8
  wires:
  delay_8/1 -> out8/1
  in8/1 -> delay_8/1
  delay_7/1 -> out7/1
  in7/1 -> delay_7/1
  delay_6/1 -> out6/1
  in6/1 -> delay_6/1
  delay_5/1 -> out5/1
  in5/1 -> delay_5/1
  delay_4/1 -> out4/1
  in4/1 -> delay_4/1
  delay_3/1 -> out3/1
  in3/1 -> delay_3/1
  delay_2/1 -> out2/1
  in2/1 -> delay_2/1
  delay_1/1 -> out1/1
  in1/1 -> delay_1/1

== system testDirect/fft_direct/bulk_delay_1_1 ==
  in1 : Inport
  in2 : Inport  Port=2
  in3 : Inport  Port=3
  in4 : Inport  Port=4
  in5 : Inport  Port=5
  in6 : Inport  Port=6
  in7 : Inport  Port=7
  in8 : Inport  Port=8
  delay_1 : xbsIndex_r4/Delay  en=off  latency=3  dbl_ovrd=off  reg_retiming=off  block_type=delay
  delay_2 : xbsIndex_r4/Delay  en=off  latency=2  dbl_ovrd=off  reg_retiming=off  block_type=delay
  delay_3 : xbsIndex_r4/Delay  en=off  latency=3  dbl_ovrd=off  reg_retiming=off  block_type=delay
  delay_4 : xbsIndex_r4/Delay  en=off  latency=2  dbl_ovrd=off  reg_retiming=off  block_type=delay
  delay_5 : xbsIndex_r4/Delay  en=off  latency=1  dbl_ovrd=off  reg_retiming=off  block_type=delay
  delay_7 : xbsIndex_r4/Delay  en=off  latency=1  dbl_ovrd=off  reg_retiming=off  block_type=delay
  out1 : Outport
  out2 : Outport  Port=2
  out3 : Outport  Port=3
  out4 : Outport  Port=4
  out5 : Outport  Port=5
  out6 : Outport  Port=6
  out7 : Outport  Port=7
  out8 : Outport  Port=8
  wires:
  in8/1 -> out8/1
  delay_7/1 -> out7/1
  in7/1 -> delay_7/1
  in6/1 -> out6/1
  delay_5/1 -> out5/1
  in5/1 -> delay_5/1
  delay_4/1 -> out4/1
  in4/1 -> delay_4/1
  delay_3/1 -> out3/1
  in3/1 -> delay_3/1
  delay_2/1 -> out2/1
  in2/1 -> delay_2/1
  delay_1/1 -> out1/1
  in1/1 -> delay_1/1

== system testDirect/fft_direct/bulk_delay_2_1 ==
  in1 : Inport
  in2 : Inport  Port=2
  in3 : Inport  Port=3
  in4 : Inport  Port=4
  in5 : Inport  Port=5
  in6 : Inport  Port=6
  in7 : Inport  Port=7
  in8 : Inport  Port=8
  delay_1 : xbsIndex_r4/Delay  en=off  latency=3  dbl_ovrd=off  reg_retiming=off  block_type=delay
  delay_2 : xbsIndex_r4/Delay  en=off  latency=2  dbl_ovrd=off  reg_retiming=off  block_type=delay
  delay_3 : xbsIndex_r4/Delay  en=off  latency=1  dbl_ovrd=off  reg_retiming=off  block_type=delay
  delay_5 : xbsIndex_r4/Delay  en=off  latency=3  dbl_ovrd=off  reg_retiming=off  block_type=delay
  delay_6 : xbsIndex_r4/Delay  en=off  latency=2  dbl_ovrd=off  reg_retiming=off  block_type=delay
  delay_7 : xbsIndex_r4/Delay  en=off  latency=1  dbl_ovrd=off  reg_retiming=off  block_type=delay
  out1 : Outport
  out2 : Outport  Port=2
  out3 : Outport  Port=3
  out4 : Outport  Port=4
  out5 : Outport  Port=5
  out6 : Outport  Port=6
  out7 : Outport  Port=7
  out8 : Outport  Port=8
  wires:
  in8/1 -> out8/1
  delay_7/1 -> out7/1
  in7/1 -> delay_7/1
  delay_6/1 -> out6/1
  in6/1 -> delay_6/1
  delay_5/1 -> out5/1
  in5/1 -> delay_5/1
  in4/1 -> out4/1
  delay_3/1 -> out3/1
  in3/1 -> delay_3/1
  delay_2/1 -> out2/1
  in2/1 -> delay_2/1
  delay_1/1 -> out1/1
  in1/1 -> delay_1/1

== system testDirect/fft_direct/bulk_delay_3_1 ==
  in1 : Inport
  in2 : Inport  Port=2
  in3 : Inport  Port=3
  in4 : Inport  Port=4
  in5 : Inport  Port=5
  in6 : Inport  Port=6
  in7 : Inport  Port=7
  in8 : Inport  Port=8
  delay_{1..4} (4 alike) : xbsIndex_r4/Delay  en=off  latency=2  dbl_ovrd=off  reg_retiming=off  block_type=delay
  out1 : Outport
  out2 : Outport  Port=2
  out3 : Outport  Port=3
  out4 : Outport  Port=4
  out5 : Outport  Port=5
  out6 : Outport  Port=6
  out7 : Outport  Port=7
  out8 : Outport  Port=8
  wires:
  in8/1 -> out8/1
  in7/1 -> out7/1
  in6/1 -> out6/1
  in5/1 -> out5/1
  delay_4/1 -> out4/1
  in4/1 -> delay_4/1
  delay_3/1 -> out3/1
  in3/1 -> delay_3/1
  delay_2/1 -> out2/1
  in2/1 -> delay_2/1
  delay_1/1 -> out1/1
  in1/1 -> delay_1/1
